simulink model "PrkgClimaActvMgr"  (R2022b)
settings: Solver=FixedStepDiscrete  StartTime=0.0  StopTime=10.0  FixedStep=0.2  RelTol=1e-3
(part 4 of 4)

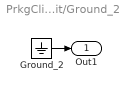
== system PrkgClimaActvMgr/PrkgClimaActvMgr_Init/Ground_2 ==
  Ground_2 : Ground
  Out1 : Outport  OutDataTypeStr=Bus: ExtVentTempStsFrmClimaGrp  PortDimensions=1
  wires:
  Ground_2/1 -> Out1/1

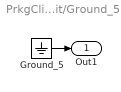
== system PrkgClimaActvMgr/PrkgClimaActvMgr_Init/Ground_5 ==
  Ground_5 : Ground
  Out1 : Outport  OutDataTypeStr=Bus: MemRecForPrkgClimaActvMgr  PortDimensions=1
  wires:
  Ground_5/1 -> Out1/1

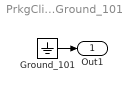
== system PrkgClimaActvMgr/PrkgClimaActvMgr_Runnable_sys/Ground_101 ==
  Ground_101 : Ground
  Out1 : Outport  OutDataTypeStr=Bus: MemRecForPrkgClimaActvMgr  PortDimensions=1
  wires:
  Ground_101/1 -> Out1/1

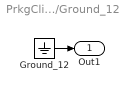
== system PrkgClimaActvMgr/PrkgClimaActvMgr_Runnable_sys/Ground_12 ==
  Ground_12 : Ground
  Out1 : Outport  OutDataTypeStr=Bus: RemSynchrnztnLcl  PortDimensions=1
  wires:
  Ground_12/1 -> Out1/1

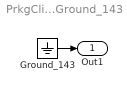
== system PrkgClimaActvMgr/PrkgClimaActvMgr_Runnable_sys/Ground_143 ==
  Ground_143 : Ground
  Out1 : Outport  OutDataTypeStr=Bus: HmiSeatClima  PortDimensions=1
  wires:
  Ground_143/1 -> Out1/1

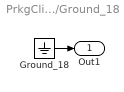
== system PrkgClimaActvMgr/PrkgClimaActvMgr_Runnable_sys/Ground_18 ==
  Ground_18 : Ground
  Out1 : Outport  OutDataTypeStr=Bus: MemRecForBlwAftRun  PortDimensions=1
  wires:
  Ground_18/1 -> Out1/1

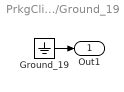
== system PrkgClimaActvMgr/PrkgClimaActvMgr_Runnable_sys/Ground_19 ==
  Ground_19 : Ground
  Out1 : Outport  OutDataTypeStr=Bus: MemRecForHighTempCln  PortDimensions=1
  wires:
  Ground_19/1 -> Out1/1

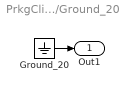
== system PrkgClimaActvMgr/PrkgClimaActvMgr_Runnable_sys/Ground_20 ==
  Ground_20 : Ground
  Out1 : Outport  OutDataTypeStr=Bus: MemRecForHMISetEngReq  PortDimensions=1
  wires:
  Ground_20/1 -> Out1/1

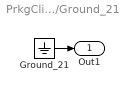
== system PrkgClimaActvMgr/PrkgClimaActvMgr_Runnable_sys/Ground_21 ==
  Ground_21 : Ground
  Out1 : Outport  OutDataTypeStr=Bus: MemRecForParkVent  PortDimensions=1
  wires:
  Ground_21/1 -> Out1/1

== system PrkgClimaActvMgr/PrkgClimaActvMgr_Runnable_sys/Ground_22 ==
  Ground_22 : Ground
  Out1 : Outport  OutDataTypeStr=Bus: MemRecForParkVentCnt  PortDimensions=1
  wires:
  Ground_22/1 -> Out1/1

== system PrkgClimaActvMgr/PrkgClimaActvMgr_Runnable_sys/Ground_40 ==
  Ground_40 : Ground
  Out1 : Outport  OutDataTypeStr=Bus: RemActvSts  PortDimensions=1
  wires:
  Ground_40/1 -> Out1/1

== system PrkgClimaActvMgr/PrkgClimaActvMgr_Runnable_sys/Ground_48 ==
  Ground_48 : Ground
  Out1 : Outport  OutDataTypeStr=Bus: RemSynchrnztnLcl  PortDimensions=1
  wires:
  Ground_48/1 -> Out1/1

== system PrkgClimaActvMgr/PrkgClimaActvMgr_Runnable_sys/Ground_88 ==
  Ground_88 : Ground
  Out1 : Outport  OutDataTypeStr=Bus: DefrstReq  PortDimensions=1
  wires:
  Ground_88/1 -> Out1/1

== system PrkgClimaActvMgr/PrkgClimaActvMgr_Runnable_sys/Ground_93 ==
  Ground_93 : Ground
  Out1 : Outport  OutDataTypeStr=Bus: ExtVentTempStsFrmClimaGrp  PortDimensions=1
  wires:
  Ground_93/1 -> Out1/1
